simulink model "V2x_TC_IntersectionCollisionWarning_cs"  (Simulink 7.6)
settings: Solver=ode5  SolverMode=SingleTasking  StartTime=0.0  StopTime=7  FixedStep=1/100  RelTol=1e-3
PreLoadFcn:
  | check_NUTS_defined;
  | 
  | assignin('base',['model_closed_' bdroot],false);addpath('../includes_V2x');
InitFcn:
  | PreScanExperimentInitFcn(bdroot)
StopFcn:
  | PreScanExperimentStopFcn(bdroot)
undefined symbols (not defined in the file or in its folder): acceleration, actorID, actorType, DiscIntNeverNeededParam, GPS_alt, GPS_lat, GPS_long, intmax, lightBarInUse, messageID, Newton, publicActorType, Raphson, rotZ, sigbuilder_block, sireneInUse, trafficLightPriority, turnAdvice, velocity, x_ini_VRML, y_ini_VRML, yawRate, z_ini_VRML
libraries: simulink x71, PreScanSimulinkLibrary x62, PreScanUsefulBlocks x16, simulink_extras x2
(part 2 of 17)

== system V2x_TC_IntersectionCollisionWarning_cs/Antenna_Tx_7 ==
  Y : Inport
  Assignment : Assignment  IndexMode=Zero-based  SampleTime=TimeStep  IndexOptions=Starting index (dialog)  Indices=PortIndex  OutputSizes=[1 1]
  Data Store Read : DataStoreRead  DataStoreName=SimCoreActuators  SampleTime=TimeStep
  Data Store Write : DataStoreWrite  DataStoreName=SimCoreActuators
  wires:
  Assignment/1 -> Data Store Write/1
  Data Store Read/1 -> Assignment/1
  Y/1 -> Assignment/2

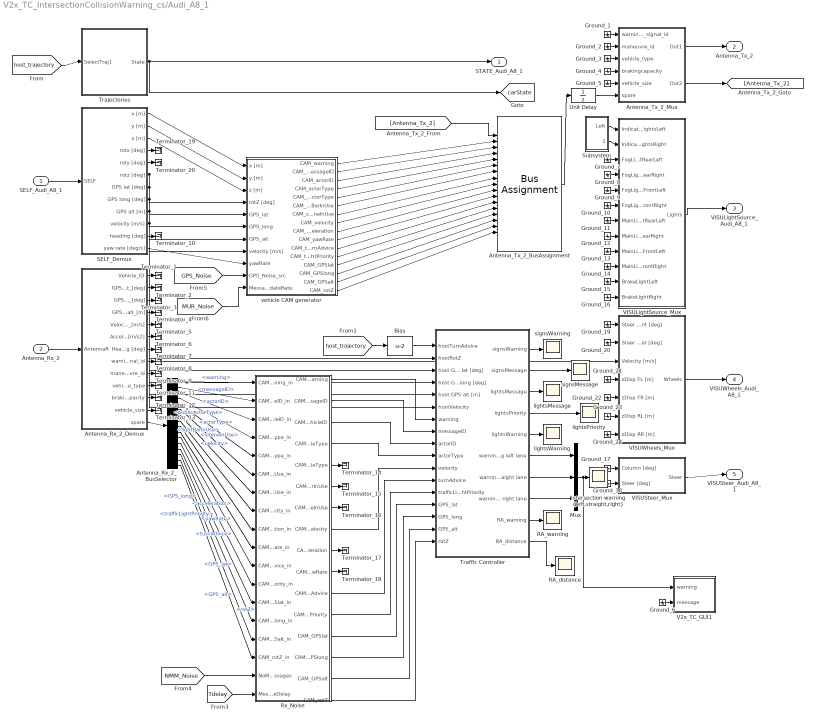
== system V2x_TC_IntersectionCollisionWarning_cs/Audi_A8_1 ==
  SELF_Audi_A8_1 : Inport  Tag=SELFInport
  Antenna_Rx_2 : Inport  Tag=AntennaRInport  Port=2
  Antenna_Rx_2_BusSelector : BusSelector  Tag=BusAntennaR  OutputSignals=warning,messageID,actorID,actorType,publicActorType,lightBarInUse,sireneInUse,velocity,acceleration,yawRate,turnAdvice,trafficLightPriority,GPS_lat,GPS_long,GPS_alt,rotZ
  Antenna_Rx_2_Demux : Subsystem  Tag=DemuxAntennaR  mask(PreScan Demux Block:){Channel=1}
      settings: DeleteFcn=PreScanBlockDeleteFcn;
      mask Display:
      | fprintf('Channel: %d',Channel)
      | port_label('output', 1, 'Vehicle_ID')
      | port_label('output', 2, 'GPS Pos Lat [deg]')
      | port_label('output', 3, 'GPS Pos Long [deg]')
      | port_label('output', 4, 'GPS Pos Alt [m]')
      | port_label('output', 5, 'Velocity [m/s]')
      | port_label('output', 6, 'Acceleration [m/s2]')
      | port_label('output', 7, 'Heading [deg]')
      | port_label('output', 8, 'Warning signal ID')
      | port_label('output', 9, 'Maneuvre ID')
      | port_label('output', 10, 'Vehicle Type')
      | port_label('output', 11, 'Braking Capacity')
      | port_label('output', 12, 'Vehicle Size')
      | port_label('output', 13, 'Spare')
  Antenna_Tx_2_BusAssignment : BusAssignment  Tag=BusAntennaT  AssignedSignals=warning,messageID,actorID,actorType,publicActorType,lightBarInUse,sireneInUse,velocity,acceleration,yawRate,turnAdvice,trafficLightPriority,GPS_lat,GPS_long,GPS_alt,rotZ
  Antenna_Tx_2_Mux : Subsystem  Tag=MuxAntennaT  mask(PreScan Mux Block:){Channel=1, TimeStep=1/100}
      mask prompts: TimeStep "Time Step [s]"
      settings: DeleteFcn=PreScanBlockDeleteFcn;
      mask Display:
      | fprintf('Channel: %d',Channel)
      | port_label('input', 1, 'Warning signal ID')
      | port_label('input', 2, 'Maneuvre ID')
      | port_label('input', 3, 'Vehicle Type')
      | port_label('input', 4, 'Braking Capacity')
      | port_label('input', 5, 'Vehicle Size')
      | port_label('input', 6, 'spare')
  Bias : Bias  Bias=-2
  Ground_{1..24} (24 alike) : Ground
  Mux : Mux  Inputs=3
  RA_distance : Scope
      settings: SaveName=ScopeData8
  RA_warning : Scope
      settings: SaveName=ScopeData7
  Rx_Noise : Subsystem
  SELF_Demux : Subsystem  Tag=DemuxSELF
      settings: DeleteFcn=PreScanBlockDeleteFcn;
      mask Type: PreScan Demux Block:
      mask Display:
      | port_label('output',1,'x [m]')
      | port_label('output',2,'y [m]')
      | port_label('output',3,'z [m]','texmode','on')
      | port_label('output',4,'Rotx [deg]','texmode','on')
      | port_label('output',5,'Roty [deg]','texmode','on')
      | port_label('output',6,'Rotz [deg]','texmode','on')
      | port_label('output',7,'GPS lat [deg]','texmode','on')
      | port_label('output',8,'GPS long [deg]','texmode','on')
      | port_label('output',9,'GPS alt [m]','texmode','on')
      | port_label('output',10,'Velocity [m/s]')
      | port_label('output',11,'Heading [deg]')
      | port_label('output',12,'Yaw rate [deg/s]')
  Subsystem : Subsystem
  Terminator_{1..20} (20 alike) : Terminator
  Traffic Controller : Subsystem
  Trajectories : Subsystem  Tag=Trajectories
      settings: DeleteFcn=PreScanBlockDeleteFcn;
      mask Type: PreScan Source Block: Trajectories
      mask Description:
      | The Trajectory block contains the trajectories defined in the PreScan Experiment Editor. The output of the trajectory block is the (vehicle) State.
      | 
      | For more information, please click Help.
      mask Help: eval('openHelp(312)')
      mask Display: image(imread('Trajectory.png'));
  Unit Delay : UnitDelay  SampleTime=-1
  V2x_TC_GUI1 : Subsystem
  VISULightSource_Mux : Subsystem  Tag=MuxLightSource
      settings: DeleteFcn=PreScanBlockDeleteFcn;
      mask Type: PreScan Mux Block:
  VISUSteer_Mux : Subsystem  Tag=MuxSteer
      settings: DeleteFcn=PreScanBlockDeleteFcn;
      mask Type: PreScan Mux Block:
  VISUWheels_Mux : Subsystem  Tag=MuxWheels  mask(PreScan Mux Block:){TimeStep=1/100}
      mask prompts: TimeStep "Auto Assigned: Time Step [s]"
      settings: DeleteFcn=PreScanBlockDeleteFcn;
  "intersection warning {left,straight,right}" : Scope
      settings: YMin=-0.1  YMax=1.1  SaveName=ScopeData5
  lightsMessage : Scope
      settings: SaveName=ScopeData2
  lightsPriority : Scope
      settings: SaveName=ScopeData3
  lightsWarning : Scope
      settings: SaveName=ScopeData4
  signsMessage : Scope
  signsWarning : Scope
  vehicle CAM generator : Subsystem
  STATE_Audi_A8_1 : Outport  Tag=StateOutport
  Antenna_Tx_2 : Outport  Tag=AntennaTOutport  Port=2
  VISULightSource_Audi_A8_1 : Outport  Tag=VisuLightsOutport  Port=3  SampleTime=1/100
  VISUWheels_Audi_A8_1 : Outport  Tag=VisuWheelsOutport  Port=4  SampleTime=1/100
  VISUSteer_Audi_A8_1 : Outport  Tag=VisuSteerOutport  Port=5  SampleTime=1/100
  tag scope: carState=global
  wires:
  [host_trajectory] -> Bias/1
  Bias/1 -> Traffic Controller/1(hostTurnAdvice)
  Mux/1 -> "intersection warning {left,straight,right}"/1, V2x_TC_GUI1/1(warning)
  Traffic Controller/1(signsWarning) -> signsWarning/1
  Traffic Controller/2(signsMessage) -> signsMessage/1
  Traffic Controller/3(lightsMessage) -> lightsMessage/1
  Traffic Controller/4(lightsPriority) -> lightsPriority/1
  Traffic Controller/5(lightsWarning) -> lightsWarning/1
  Traffic Controller/6(warning left lane) -> Mux/1
  Traffic Controller/7(warning straight lane) -> Mux/2
  Traffic Controller/8(warning right lane) -> Mux/3
  Traffic Controller/9(RA_warning) -> RA_warning/1
  Traffic Controller/10(RA_distance) -> RA_distance/1
  [NMM_Noise] -> Rx_Noise/17(NoMissedMessages)
  [Tdelay] -> Rx_Noise/18(MessageDelay)
  [GPS_Noise] -> vehicle CAM generator/10(GPS_Noise_src)
  [MUR_Noise] -> vehicle CAM generator/11(MessageUpdateRate)
  Rx_Noise/5(CAM_publicVehicleType) -> Terminator_14/1
  Rx_Noise/6(CAM_lightBarInUse) -> Terminator_15/1
  Rx_Noise/7(CAM_sireneInUse) -> Terminator_16/1
  Rx_Noise/9(CAM_acceleration) -> Terminator_17/1
  Rx_Noise/10(CAM_yawRate) -> Terminator_18/1
  Rx_Noise/1(CAM_warning) -> Traffic Controller/7(warning)
  Rx_Noise/2(CAM_messageID) -> Traffic Controller/8(messageID)
  Rx_Noise/3(CAM_vehicleID) -> Traffic Controller/9(actorID)
  Rx_Noise/4(CAM_vehicleType) -> Traffic Controller/10(actorType)
  Rx_Noise/8(CAM_velocity) -> Traffic Controller/11(velocity)
  Rx_Noise/11(CAM_turnAdvice) -> Traffic Controller/12(turnAdvice)
  Rx_Noise/12(CAM_trafficLightPriority) -> Traffic Controller/13(trafficLightPriority)
  Rx_Noise/13(CAM_GPSlat) -> Traffic Controller/14(GPS_lat)
  Rx_Noise/14(CAM_GPSlong) -> Traffic Controller/15(GPS_long)
  Rx_Noise/15(CAM_GPSalt) -> Traffic Controller/16(GPS_alt)
  Rx_Noise/16(CAM_rotZ) -> Traffic Controller/17(rotZ)
  SELF_Audi_A8_1/1 -> SELF_Demux/1(SELF)
  Antenna_Rx_2_Demux/13(spare) -> Antenna_Rx_2_BusSelector/1
  Antenna_Rx_2/1 -> Antenna_Rx_2_Demux/1(AntennaR)
  Antenna_Tx_2_Mux/2(Out2) -> [Antenna_Tx_2]
  [Antenna_Tx_2] -> Antenna_Tx_2_BusAssignment/1
  Antenna_Tx_2_Mux/1(Out1) -> Antenna_Tx_2/1
  VISULightSource_Mux/1(Lights) -> VISULightSource_Audi_A8_1/1
  VISUWheels_Mux/1(Wheels) -> VISUWheels_Audi_A8_1/1
  VISUSteer_Mux/1(Steer) -> VISUSteer_Audi_A8_1/1
  Trajectories/1(State) -> STATE_Audi_A8_1/1, [carState]
  SELF_Demux/5(roty [deg]) -> Terminator_20/1
  SELF_Demux/4(rotx [deg]) -> Terminator_19/1
  Antenna_Rx_2_Demux/12(vehicle_size) -> Terminator_13/1
  Antenna_Rx_2_Demux/11(brakingcapacity) -> Terminator_12/1
  Antenna_Rx_2_Demux/10(vehicle_type) -> Terminator_11/1
  vehicle CAM generator/16(CAM_rotZ) -> Antenna_Tx_2_BusAssignment/17
  vehicle CAM generator/15(CAM_GPSalt) -> Antenna_Tx_2_BusAssignment/16
  vehicle CAM generator/14(CAM_GPSlong) -> Antenna_Tx_2_BusAssignment/15
  vehicle CAM generator/13(CAM_GPSlat) -> Antenna_Tx_2_BusAssignment/14
  vehicle CAM generator/12(CAM_trafficLightPriority) -> Antenna_Tx_2_BusAssignment/13
  vehicle CAM generator/11(CAM_turnAdvice) -> Antenna_Tx_2_BusAssignment/12
  Antenna_Rx_2_BusSelector/16 -> Rx_Noise/16(CAM_rotZ_in)  "<rotZ>"
  Antenna_Rx_2_BusSelector/15 -> Rx_Noise/15(CAM_GPSalt_in)  "<GPS_alt>"
  Antenna_Rx_2_BusSelector/14 -> Rx_Noise/14(CAM_GPSlong_in)  "<GPS_long>"
  Antenna_Rx_2_BusSelector/13 -> Rx_Noise/13(CAM_GPSlat_in)  "<GPS_lat>"
  Antenna_Rx_2_BusSelector/12 -> Rx_Noise/12(CAM_trafficLightPriority_in)  "<trafficLightPriority>"
  Antenna_Rx_2_BusSelector/11 -> Rx_Noise/11(CAM_turnAdvice_in)  "<turnAdvice>"
  Antenna_Rx_2_BusSelector/10 -> Rx_Noise/10(CAM_yawRate_in)  "<yawRate>"
  Antenna_Rx_2_BusSelector/9 -> Rx_Noise/9(CAM_acceleration_in)  "<acceleration>"
  Antenna_Rx_2_BusSelector/8 -> Rx_Noise/8(CAM_velocity_in)  "<velocity>"
  Antenna_Rx_2_BusSelector/7 -> Rx_Noise/7(CAM_sireneInUse_in)  "<sireneInUse>"
  Antenna_Rx_2_BusSelector/6 -> Rx_Noise/6(CAM_lightBarInUse_in)  "<lightBarInUse>"
  Antenna_Rx_2_BusSelector/5 -> Rx_Noise/5(CAM_publicVehicleType_in)  "<publicActorType>"
  Antenna_Rx_2_BusSelector/4 -> Rx_Noise/4(CAM_vehicleType_in)  "<actorType>"
  Antenna_Rx_2_BusSelector/3 -> Rx_Noise/3(CAM_vehicleID_in)  "<actorID>"
  Antenna_Rx_2_BusSelector/2 -> Rx_Noise/2(CAM_messageID_in)  "<messageID>"
  Antenna_Rx_2_BusSelector/1 -> Rx_Noise/1(CAM_warning_in)  "<warning>"
  [host_trajectory] -> Trajectories/1(SelectTraj1)
  SELF_Demux/10(velocity [m/s]) -> vehicle CAM generator/8(velocity [m/s]), VISUWheels_Mux/3(Velocity [m/s]), Traffic Controller/6(hostVelocity)
  SELF_Demux/9(GPS alt [m]) -> vehicle CAM generator/7(GPS_alt), Traffic Controller/5(host GPS alt [m])
  SELF_Demux/8(GPS long [deg]) -> vehicle CAM generator/6(GPS_long), Traffic Controller/4(host GPS long [deg])
  SELF_Demux/7(GPS lat [deg]) -> vehicle CAM generator/5(GPS_lat), Traffic Controller/3(host GPS lat [deg])
  SELF_Demux/1(x [m]) -> vehicle CAM generator/1(x [m])
  SELF_Demux/12(yaw rate [deg/s]) -> vehicle CAM generator/9(yawRate)
  SELF_Demux/3(z [m]) -> vehicle CAM generator/3(z [m])
  SELF_Demux/2(y [m]) -> vehicle CAM generator/2(y [m])
  SELF_Demux/6(rotz [deg]) -> vehicle CAM generator/4(rotZ [deg]), Traffic Controller/2(hostRotZ)
  vehicle CAM generator/1(CAM_warning) -> Antenna_Tx_2_BusAssignment/2
  vehicle CAM generator/2(CAM_messageID) -> Antenna_Tx_2_BusAssignment/3
  vehicle CAM generator/3(CAM_actorID) -> Antenna_Tx_2_BusAssignment/4
  vehicle CAM generator/4(CAM_actorType) -> Antenna_Tx_2_BusAssignment/5
  vehicle CAM generator/5(CAM_publicActorType) -> Antenna_Tx_2_BusAssignment/6
  vehicle CAM generator/6(CAM_lightBarInUse) -> Antenna_Tx_2_BusAssignment/7
  vehicle CAM generator/7(CAM_sireneInUse) -> Antenna_Tx_2_BusAssignment/8
  vehicle CAM generator/8(CAM_velocity) -> Antenna_Tx_2_BusAssignment/9
  vehicle CAM generator/9(CAM_acceleration) -> Antenna_Tx_2_BusAssignment/10
  vehicle CAM generator/10(CAM_yawRate) -> Antenna_Tx_2_BusAssignment/11
  Subsystem/1(Left) -> VISULightSource_Mux/1(IndicatorLightsLeft)
  Subsystem/2(Right) -> VISULightSource_Mux/2(IndicatorLightsRight)
  Unit Delay/1 -> Antenna_Tx_2_Mux/6(spare)
  Antenna_Tx_2_BusAssignment/1 -> Unit Delay/1
  Antenna_Rx_2_Demux/1(Vehicle_ID) -> Terminator_1/1
  Antenna_Rx_2_Demux/2(GPS_pos_lat_[deg]) -> Terminator_2/1
  Antenna_Rx_2_Demux/3(GPS_pos_long_[deg]) -> Terminator_3/1
  Antenna_Rx_2_Demux/4(GPS_pos_alt_[m]) -> Terminator_4/1
  Antenna_Rx_2_Demux/5(Velocity_[m/s]) -> Terminator_5/1
  Antenna_Rx_2_Demux/6(Acceleration_[m/s2]) -> Terminator_6/1
  Antenna_Rx_2_Demux/7(Heading [deg]) -> Terminator_7/1
  Antenna_Rx_2_Demux/8(warning_signal_id) -> Terminator_8/1
  Antenna_Rx_2_Demux/9(maneuvre_id) -> Terminator_9/1
  Ground_1/1 -> Antenna_Tx_2_Mux/1(warning_signal_id)
  Ground_2/1 -> Antenna_Tx_2_Mux/2(maneuvre_id)
  Ground_3/1 -> Antenna_Tx_2_Mux/3(vehicle_type)
  Ground_4/1 -> Antenna_Tx_2_Mux/4(brakingcapacity)
  Ground_5/1 -> Antenna_Tx_2_Mux/5(vehicle_size)
  SELF_Demux/11(heading [deg]) -> Terminator_10/1
  Ground_6/1 -> V2x_TC_GUI1/2(message)
  Ground_7/1 -> VISULightSource_Mux/3(FogLightRearLeft)
  Ground_8/1 -> VISULightSource_Mux/4(FogLightRearRight)
  Ground_9/1 -> VISULightSource_Mux/5(FogLightFrontLeft)
  Ground_10/1 -> VISULightSource_Mux/6(FogLightFrontRight)
  Ground_11/1 -> VISULightSource_Mux/7(MainLightRearLeft)
  Ground_12/1 -> VISULightSource_Mux/8(MainLightRearRight)
  Ground_13/1 -> VISULightSource_Mux/9(MainLightFrontLeft)
  Ground_14/1 -> VISULightSource_Mux/10(MainLightFrontRight)
  Ground_15/1 -> VISULightSource_Mux/11(BrakeLightLeft)
  Ground_16/1 -> VISULightSource_Mux/12(BrakeLightRight)
  Ground_17/1 -> VISUSteer_Mux/1(Column [deg])
  Ground_18/1 -> VISUSteer_Mux/2(Steer [deg])
  Ground_19/1 -> VISUWheels_Mux/1(Steer Angle Front [deg])
  Ground_20/1 -> VISUWheels_Mux/2(Steer Angle Rear [deg])
  Ground_21/1 -> VISUWheels_Mux/4(zDisp FL [m])
  Ground_22/1 -> VISUWheels_Mux/5(zDisp FR [m])
  Ground_23/1 -> VISUWheels_Mux/6(zDisp RL [m])
  Ground_24/1 -> VISUWheels_Mux/7(zDisp RR [m])

== system V2x_TC_IntersectionCollisionWarning_cs/Citroen_C3_1 ==
  SELF_Citroen_C3_1 : Inport  Tag=SELFInport
  Dynamics_Empty : Subsystem  Tag=Dynamics_Empty
      mask Display: port_label('output', 1, 'State');text(0,0,' Empty\n')
  SELF_Demux : Subsystem  Tag=DemuxSELF
      settings: DeleteFcn=PreScanBlockDeleteFcn;
      mask Type: PreScan Demux Block:
      mask Display:
      | port_label('output',1,'x [m]')
      | port_label('output',2,'y [m]')
      | port_label('output',3,'z [m]','texmode','on')
      | port_label('output',4,'Rotx [deg]','texmode','on')
      | port_label('output',5,'Roty [deg]','texmode','on')
      | port_label('output',6,'Rotz [deg]','texmode','on')
      | port_label('output',7,'GPS lat [deg]','texmode','on')
      | port_label('output',8,'GPS long [deg]','texmode','on')
      | port_label('output',9,'GPS alt [m]','texmode','on')
      | port_label('output',10,'Velocity [m/s]')
      | port_label('output',11,'Heading [deg]')
      | port_label('output',12,'Yaw rate [deg/s]')
  Terminator_{1..12} (12 alike) : Terminator
  STATE_Citroen_C3_1 : Outport  Tag=StateOutport
  wires:
  SELF_Citroen_C3_1/1 -> SELF_Demux/1(SELF)
  Dynamics_Empty/1(State) -> STATE_Citroen_C3_1/1
  SELF_Demux/1(x [m]) -> Terminator_1/1
  SELF_Demux/2(y [m]) -> Terminator_2/1
  SELF_Demux/3(z [m]) -> Terminator_3/1
  SELF_Demux/4(rotx [deg]) -> Terminator_4/1
  SELF_Demux/5(roty [deg]) -> Terminator_5/1
  SELF_Demux/6(rotz [deg]) -> Terminator_6/1
  SELF_Demux/7(GPS lat [deg]) -> Terminator_7/1
  SELF_Demux/8(GPS long [deg]) -> Terminator_8/1
  SELF_Demux/9(GPS alt [m]) -> Terminator_9/1
  SELF_Demux/10(velocity [m/s]) -> Terminator_10/1
  SELF_Demux/11(heading [deg]) -> Terminator_11/1
  SELF_Demux/12(yaw rate [deg/s]) -> Terminator_12/1

== system V2x_TC_IntersectionCollisionWarning_cs/Female_Regular_wBuggy_1 ==
  SELF_Female_Regular_wBuggy_1 : Inport  Tag=SELFInport
  Dynamics_Empty : Subsystem  Tag=Dynamics_Empty
      mask Display: port_label('output', 1, 'State');text(0,0,' Empty\n')
  SELF_Demux : Subsystem  Tag=DemuxSELF
      settings: DeleteFcn=PreScanBlockDeleteFcn;
      mask Type: PreScan Demux Block:
      mask Display:
      | port_label('output',1,'x [m]')
      | port_label('output',2,'y [m]')
      | port_label('output',3,'z [m]','texmode','on')
      | port_label('output',4,'Rotx [deg]','texmode','on')
      | port_label('output',5,'Roty [deg]','texmode','on')
      | port_label('output',6,'Rotz [deg]','texmode','on')
      | port_label('output',7,'GPS lat [deg]','texmode','on')
      | port_label('output',8,'GPS long [deg]','texmode','on')
      | port_label('output',9,'GPS alt [m]','texmode','on')
      | port_label('output',10,'Velocity [m/s]')
      | port_label('output',11,'Heading [deg]')
      | port_label('output',12,'Yaw rate [deg/s]')
  Terminator_{1..12} (12 alike) : Terminator
  STATE_Female_Regular_wBuggy_1 : Outport  Tag=StateOutport
  wires:
  SELF_Female_Regular_wBuggy_1/1 -> SELF_Demux/1(SELF)
  Dynamics_Empty/1(State) -> STATE_Female_Regular_wBuggy_1/1
  SELF_Demux/1(x [m]) -> Terminator_1/1
  SELF_Demux/2(y [m]) -> Terminator_2/1
  SELF_Demux/3(z [m]) -> Terminator_3/1
  SELF_Demux/4(rotx [deg]) -> Terminator_4/1
  SELF_Demux/5(roty [deg]) -> Terminator_5/1
  SELF_Demux/6(rotz [deg]) -> Terminator_6/1
  SELF_Demux/7(GPS lat [deg]) -> Terminator_7/1
  SELF_Demux/8(GPS long [deg]) -> Terminator_8/1
  SELF_Demux/9(GPS alt [m]) -> Terminator_9/1
  SELF_Demux/10(velocity [m/s]) -> Terminator_10/1
  SELF_Demux/11(heading [deg]) -> Terminator_11/1
  SELF_Demux/12(yaw rate [deg/s]) -> Terminator_12/1

== system V2x_TC_IntersectionCollisionWarning_cs/Fiat_Bravo_1 ==
  SELF_Fiat_Bravo_1 : Inport  Tag=SELFInport
  Antenna_Rx_3 : Inport  Tag=AntennaRInport  Port=2
  Antenna_Rx_3_BusSelector : BusSelector  Tag=BusAntennaR  OutputSignals=warning,messageID,actorID,actorType,publicActorType,lightBarInUse,sireneInUse,velocity,acceleration,yawRate
  Antenna_Rx_3_Demux : Subsystem  Tag=DemuxAntennaR  mask(PreScan Demux Block:){Channel=1}
      settings: DeleteFcn=PreScanBlockDeleteFcn;
      mask Display:
      | fprintf('Channel: %d',Channel)
      | port_label('output', 1, 'Vehicle_ID')
      | port_label('output', 2, 'GPS Pos Lat [deg]')
      | port_label('output', 3, 'GPS Pos Long [deg]')
      | port_label('output', 4, 'GPS Pos Alt [m]')
      | port_label('output', 5, 'Velocity [m/s]')
      | port_label('output', 6, 'Acceleration [m/s2]')
      | port_label('output', 7, 'Heading [deg]')
      | port_label('output', 8, 'Warning signal ID')
      | port_label('output', 9, 'Maneuvre ID')
      | port_label('output', 10, 'Vehicle Type')
      | port_label('output', 11, 'Braking Capacity')
      | port_label('output', 12, 'Vehicle Size')
      | port_label('output', 13, 'Spare')
  Antenna_Tx_4_BusAssignment : BusAssignment  Tag=BusAntennaT  AssignedSignals=warning,messageID,actorID,actorType,publicActorType,lightBarInUse,sireneInUse,velocity,acceleration,yawRate,turnAdvice,trafficLightPriority,GPS_lat,GPS_long,GPS_alt,rotZ
  Antenna_Tx_4_Mux : Subsystem  Tag=MuxAntennaT  mask(PreScan Mux Block:){Channel=1, TimeStep=1/100}
      mask prompts: TimeStep "Time Step [s]"
      settings: DeleteFcn=PreScanBlockDeleteFcn;
      mask Display:
      | fprintf('Channel: %d',Channel)
      | port_label('input', 1, 'Warning signal ID')
      | port_label('input', 2, 'Maneuvre ID')
      | port_label('input', 3, 'Vehicle Type')
      | port_label('input', 4, 'Braking Capacity')
      | port_label('input', 5, 'Vehicle Size')
      | port_label('input', 6, 'spare')
  Dynamics_Empty : Subsystem  Tag=Dynamics_Empty
      mask Display: port_label('output', 1, 'State');text(0,0,' Empty\n')
  Ground_{1..5} (5 alike) : Ground
  SELF_Demux : Subsystem  Tag=DemuxSELF
      settings: DeleteFcn=PreScanBlockDeleteFcn;
      mask Type: PreScan Demux Block:
      mask Display:
      | port_label('output',1,'x [m]')
      | port_label('output',2,'y [m]')
      | port_label('output',3,'z [m]','texmode','on')
      | port_label('output',4,'Rotx [deg]','texmode','on')
      | port_label('output',5,'Roty [deg]','texmode','on')
      | port_label('output',6,'Rotz [deg]','texmode','on')
      | port_label('output',7,'GPS lat [deg]','texmode','on')
      | port_label('output',8,'GPS long [deg]','texmode','on')
      | port_label('output',9,'GPS alt [m]','texmode','on')
      | port_label('output',10,'Velocity [m/s]')
      | port_label('output',11,'Heading [deg]')
      | port_label('output',12,'Yaw rate [deg/s]')
  Terminator_{1..25} (25 alike) : Terminator
  Unit Delay : UnitDelay  SampleTime=-1
  vehicle CAM generator : Subsystem
  STATE_Fiat_Bravo_1 : Outport  Tag=StateOutport
  Antenna_Tx_4 : Outport  Tag=AntennaTOutport  Port=2
  wires:
  [GPS_Noise] -> vehicle CAM generator/10(GPS_Noise_src)
  [MUR_Noise] -> vehicle CAM generator/11(MessageUpdateRate)
  SELF_Fiat_Bravo_1/1 -> SELF_Demux/1(SELF)
  Antenna_Rx_3_Demux/13(spare) -> Antenna_Rx_3_BusSelector/1
  Antenna_Rx_3/1 -> Antenna_Rx_3_Demux/1(AntennaR)
  Antenna_Tx_4_Mux/2(Out2) -> [Antenna_Tx_4]
  [Antenna_Tx_4] -> Antenna_Tx_4_BusAssignment/1
  Antenna_Tx_4_Mux/1(Out1) -> Antenna_Tx_4/1
  Dynamics_Empty/1(State) -> STATE_Fiat_Bravo_1/1
  vehicle CAM generator/16(CAM_rotZ) -> Antenna_Tx_4_BusAssignment/17
  vehicle CAM generator/15(CAM_GPSalt) -> Antenna_Tx_4_BusAssignment/16
  vehicle CAM generator/14(CAM_GPSlong) -> Antenna_Tx_4_BusAssignment/15
  vehicle CAM generator/13(CAM_GPSlat) -> Antenna_Tx_4_BusAssignment/14
  vehicle CAM generator/12(CAM_trafficLightPriority) -> Antenna_Tx_4_BusAssignment/13
  vehicle CAM generator/11(CAM_turnAdvice) -> Antenna_Tx_4_BusAssignment/12
  SELF_Demux/12(yaw rate [deg/s]) -> vehicle CAM generator/9(yawRate)
  SELF_Demux/10(velocity [m/s]) -> vehicle CAM generator/8(velocity [m/s])
  SELF_Demux/9(GPS alt [m]) -> vehicle CAM generator/7(GPS_alt)
  SELF_Demux/8(GPS long [deg]) -> vehicle CAM generator/6(GPS_long)
  SELF_Demux/7(GPS lat [deg]) -> vehicle CAM generator/5(GPS_lat)
  SELF_Demux/3(z [m]) -> vehicle CAM generator/3(z [m])
  SELF_Demux/2(y [m]) -> vehicle CAM generator/2(y [m])
  SELF_Demux/1(x [m]) -> vehicle CAM generator/1(x [m])
  SELF_Demux/6(rotz [deg]) -> vehicle CAM generator/4(rotZ [deg])
  vehicle CAM generator/1(CAM_warning) -> Antenna_Tx_4_BusAssignment/2
  vehicle CAM generator/2(CAM_messageID) -> Antenna_Tx_4_BusAssignment/3
  vehicle CAM generator/3(CAM_actorID) -> Antenna_Tx_4_BusAssignment/4
  vehicle CAM generator/4(CAM_actorType) -> Antenna_Tx_4_BusAssignment/5
  vehicle CAM generator/5(CAM_publicActorType) -> Antenna_Tx_4_BusAssignment/6
  vehicle CAM generator/6(CAM_lightBarInUse) -> Antenna_Tx_4_BusAssignment/7
  vehicle CAM generator/7(CAM_sireneInUse) -> Antenna_Tx_4_BusAssignment/8
  vehicle CAM generator/8(CAM_velocity) -> Antenna_Tx_4_BusAssignment/9
  vehicle CAM generator/9(CAM_acceleration) -> Antenna_Tx_4_BusAssignment/10
  vehicle CAM generator/10(CAM_yawRate) -> Antenna_Tx_4_BusAssignment/11
  Antenna_Rx_3_BusSelector/1 -> Terminator_16/1  "<warning>"
  Antenna_Rx_3_BusSelector/2 -> Terminator_17/1  "<messageID>"
  Antenna_Rx_3_BusSelector/3 -> Terminator_18/1  "<actorID>"
  Antenna_Rx_3_BusSelector/4 -> Terminator_19/1  "<actorType>"
  Antenna_Rx_3_BusSelector/5 -> Terminator_20/1  "<publicActorType>"
  Antenna_Rx_3_BusSelector/6 -> Terminator_21/1  "<lightBarInUse>"
  Antenna_Rx_3_BusSelector/7 -> Terminator_22/1  "<sireneInUse>"
  Antenna_Rx_3_BusSelector/8 -> Terminator_23/1  "<velocity>"
  Antenna_Rx_3_BusSelector/9 -> Terminator_24/1  "<acceleration>"
  Antenna_Rx_3_BusSelector/10 -> Terminator_25/1  "<yawRate>"
  Unit Delay/1 -> Antenna_Tx_4_Mux/6(spare)
  Antenna_Tx_4_BusAssignment/1 -> Unit Delay/1
  Antenna_Rx_3_Demux/1(Vehicle_ID) -> Terminator_1/1
  Antenna_Rx_3_Demux/2(GPS_pos_lat_[deg]) -> Terminator_2/1
  Antenna_Rx_3_Demux/3(GPS_pos_long_[deg]) -> Terminator_3/1
  Antenna_Rx_3_Demux/4(GPS_pos_alt_[m]) -> Terminator_4/1
  Antenna_Rx_3_Demux/5(Velocity_[m/s]) -> Terminator_5/1
  Antenna_Rx_3_Demux/6(Acceleration_[m/s2]) -> Terminator_6/1
  Antenna_Rx_3_Demux/7(Heading [deg]) -> Terminator_7/1
  Antenna_Rx_3_Demux/8(warning_signal_id) -> Terminator_8/1
  Antenna_Rx_3_Demux/9(maneuvre_id) -> Terminator_9/1
  Antenna_Rx_3_Demux/10(vehicle_type) -> Terminator_10/1
  Antenna_Rx_3_Demux/11(brakingcapacity) -> Terminator_11/1
  Antenna_Rx_3_Demux/12(vehicle_size) -> Terminator_12/1
  Ground_1/1 -> Antenna_Tx_4_Mux/1(warning_signal_id)
  Ground_2/1 -> Antenna_Tx_4_Mux/2(maneuvre_id)
  Ground_3/1 -> Antenna_Tx_4_Mux/3(vehicle_type)
  Ground_4/1 -> Antenna_Tx_4_Mux/4(brakingcapacity)
  Ground_5/1 -> Antenna_Tx_4_Mux/5(vehicle_size)
  SELF_Demux/4(rotx [deg]) -> Terminator_13/1
  SELF_Demux/5(roty [deg]) -> Terminator_14/1
  SELF_Demux/11(heading [deg]) -> Terminator_15/1

== system V2x_TC_IntersectionCollisionWarning_cs/Ford_Fiesta_1 ==
  SELF_Ford_Fiesta_1 : Inport  Tag=SELFInport
  Dynamics_Empty : Subsystem  Tag=Dynamics_Empty
      mask Display: port_label('output', 1, 'State');text(0,0,' Empty\n')
  SELF_Demux : Subsystem  Tag=DemuxSELF
      settings: DeleteFcn=PreScanBlockDeleteFcn;
      mask Type: PreScan Demux Block:
      mask Display:
      | port_label('output',1,'x [m]')
      | port_label('output',2,'y [m]')
      | port_label('output',3,'z [m]','texmode','on')
      | port_label('output',4,'Rotx [deg]','texmode','on')
      | port_label('output',5,'Roty [deg]','texmode','on')
      | port_label('output',6,'Rotz [deg]','texmode','on')
      | port_label('output',7,'GPS lat [deg]','texmode','on')
      | port_label('output',8,'GPS long [deg]','texmode','on')
      | port_label('output',9,'GPS alt [m]','texmode','on')
      | port_label('output',10,'Velocity [m/s]')
      | port_label('output',11,'Heading [deg]')
      | port_label('output',12,'Yaw rate [deg/s]')
  Terminator_{1..12} (12 alike) : Terminator
  STATE_Ford_Fiesta_1 : Outport  Tag=StateOutport
  wires:
  SELF_Ford_Fiesta_1/1 -> SELF_Demux/1(SELF)
  Dynamics_Empty/1(State) -> STATE_Ford_Fiesta_1/1
  SELF_Demux/1(x [m]) -> Terminator_1/1
  SELF_Demux/2(y [m]) -> Terminator_2/1
  SELF_Demux/3(z [m]) -> Terminator_3/1
  SELF_Demux/4(rotx [deg]) -> Terminator_4/1
  SELF_Demux/5(roty [deg]) -> Terminator_5/1
  SELF_Demux/6(rotz [deg]) -> Terminator_6/1
  SELF_Demux/7(GPS lat [deg]) -> Terminator_7/1
  SELF_Demux/8(GPS long [deg]) -> Terminator_8/1
  SELF_Demux/9(GPS alt [m]) -> Terminator_9/1
  SELF_Demux/10(velocity [m/s]) -> Terminator_10/1
  SELF_Demux/11(heading [deg]) -> Terminator_11/1
  SELF_Demux/12(yaw rate [deg/s]) -> Terminator_12/1

== system V2x_TC_IntersectionCollisionWarning_cs/Ford_FocusWagon_1 ==
  SELF_Ford_FocusWagon_1 : Inport  Tag=SELFInport
  Dynamics_Empty : Subsystem  Tag=Dynamics_Empty
      mask Display: port_label('output', 1, 'State');text(0,0,' Empty\n')
  SELF_Demux : Subsystem  Tag=DemuxSELF
      settings: DeleteFcn=PreScanBlockDeleteFcn;
      mask Type: PreScan Demux Block:
      mask Display:
      | port_label('output',1,'x [m]')
      | port_label('output',2,'y [m]')
      | port_label('output',3,'z [m]','texmode','on')
      | port_label('output',4,'Rotx [deg]','texmode','on')
      | port_label('output',5,'Roty [deg]','texmode','on')
      | port_label('output',6,'Rotz [deg]','texmode','on')
      | port_label('output',7,'GPS lat [deg]','texmode','on')
      | port_label('output',8,'GPS long [deg]','texmode','on')
      | port_label('output',9,'GPS alt [m]','texmode','on')
      | port_label('output',10,'Velocity [m/s]')
      | port_label('output',11,'Heading [deg]')
      | port_label('output',12,'Yaw rate [deg/s]')
  Terminator_{1..12} (12 alike) : Terminator
  STATE_Ford_FocusWagon_1 : Outport  Tag=StateOutport
  wires:
  SELF_Ford_FocusWagon_1/1 -> SELF_Demux/1(SELF)
  Dynamics_Empty/1(State) -> STATE_Ford_FocusWagon_1/1
  SELF_Demux/1(x [m]) -> Terminator_1/1
  SELF_Demux/2(y [m]) -> Terminator_2/1
  SELF_Demux/3(z [m]) -> Terminator_3/1
  SELF_Demux/4(rotx [deg]) -> Terminator_4/1
  SELF_Demux/5(roty [deg]) -> Terminator_5/1
  SELF_Demux/6(rotz [deg]) -> Terminator_6/1
  SELF_Demux/7(GPS lat [deg]) -> Terminator_7/1
  SELF_Demux/8(GPS long [deg]) -> Terminator_8/1
  SELF_Demux/9(GPS alt [m]) -> Terminator_9/1
  SELF_Demux/10(velocity [m/s]) -> Terminator_10/1
  SELF_Demux/11(heading [deg]) -> Terminator_11/1
  SELF_Demux/12(yaw rate [deg/s]) -> Terminator_12/1

== system V2x_TC_IntersectionCollisionWarning_cs/GetSensors ==
  dummy : Inport
  GetSensors : M-S-Function  FunctionName=GetSensorsBlock  Parameters=TimeStep NrOfSensors
  tag scope: SimCoreSensors=global
  wires:
  GetSensors/1 -> [SimCoreSensors]
  dummy/1 -> GetSensors/1

== system V2x_TC_IntersectionCollisionWarning_cs/Male_Regular_wSuitcase_1 ==
  SELF_Male_Regular_wSuitcase_1 : Inport  Tag=SELFInport
  Dynamics_Empty : Subsystem  Tag=Dynamics_Empty
      mask Display: port_label('output', 1, 'State');text(0,0,' Empty\n')
  SELF_Demux : Subsystem  Tag=DemuxSELF
      settings: DeleteFcn=PreScanBlockDeleteFcn;
      mask Type: PreScan Demux Block:
      mask Display:
      | port_label('output',1,'x [m]')
      | port_label('output',2,'y [m]')
      | port_label('output',3,'z [m]','texmode','on')
      | port_label('output',4,'Rotx [deg]','texmode','on')
      | port_label('output',5,'Roty [deg]','texmode','on')
      | port_label('output',6,'Rotz [deg]','texmode','on')
      | port_label('output',7,'GPS lat [deg]','texmode','on')
      | port_label('output',8,'GPS long [deg]','texmode','on')
      | port_label('output',9,'GPS alt [m]','texmode','on')
      | port_label('output',10,'Velocity [m/s]')
      | port_label('output',11,'Heading [deg]')
      | port_label('output',12,'Yaw rate [deg/s]')
  Terminator_{1..12} (12 alike) : Terminator
  STATE_Male_Regular_wSuitcase_1 : Outport  Tag=StateOutport
  wires:
  SELF_Male_Regular_wSuitcase_1/1 -> SELF_Demux/1(SELF)
  Dynamics_Empty/1(State) -> STATE_Male_Regular_wSuitcase_1/1
  SELF_Demux/1(x [m]) -> Terminator_1/1
  SELF_Demux/2(y [m]) -> Terminator_2/1
  SELF_Demux/3(z [m]) -> Terminator_3/1
  SELF_Demux/4(rotx [deg]) -> Terminator_4/1
  SELF_Demux/5(roty [deg]) -> Terminator_5/1
  SELF_Demux/6(rotz [deg]) -> Terminator_6/1
  SELF_Demux/7(GPS lat [deg]) -> Terminator_7/1
  SELF_Demux/8(GPS long [deg]) -> Terminator_8/1
  SELF_Demux/9(GPS alt [m]) -> Terminator_9/1
  SELF_Demux/10(velocity [m/s]) -> Terminator_10/1
  SELF_Demux/11(heading [deg]) -> Terminator_11/1
  SELF_Demux/12(yaw rate [deg/s]) -> Terminator_12/1

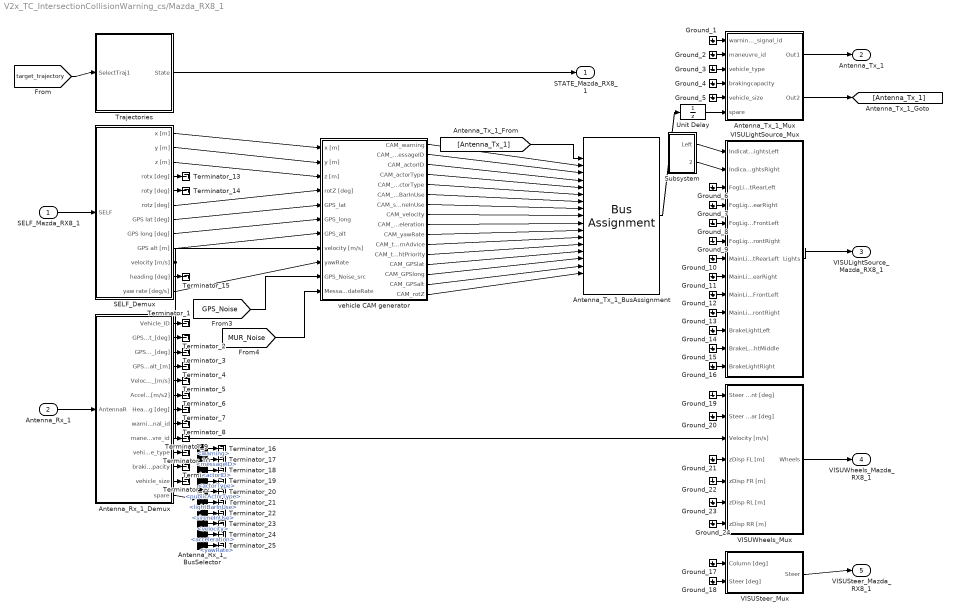
== system V2x_TC_IntersectionCollisionWarning_cs/Mazda_RX8_1 ==
  SELF_Mazda_RX8_1 : Inport  Tag=SELFInport
  Antenna_Rx_1 : Inport  Tag=AntennaRInport  Port=2
  Antenna_Rx_1_BusSelector : BusSelector  Tag=BusAntennaR  OutputSignals=warning,messageID,actorID,actorType,publicActorType,lightBarInUse,sireneInUse,velocity,acceleration,yawRate
  Antenna_Rx_1_Demux : Subsystem  Tag=DemuxAntennaR  mask(PreScan Demux Block:){Channel=1}
      settings: DeleteFcn=PreScanBlockDeleteFcn;
      mask Display:
      | fprintf('Channel: %d',Channel)
      | port_label('output', 1, 'Vehicle_ID')
      | port_label('output', 2, 'GPS Pos Lat [deg]')
      | port_label('output', 3, 'GPS Pos Long [deg]')
      | port_label('output', 4, 'GPS Pos Alt [m]')
      | port_label('output', 5, 'Velocity [m/s]')
      | port_label('output', 6, 'Acceleration [m/s2]')
      | port_label('output', 7, 'Heading [deg]')
      | port_label('output', 8, 'Warning signal ID')
      | port_label('output', 9, 'Maneuvre ID')
      | port_label('output', 10, 'Vehicle Type')
      | port_label('output', 11, 'Braking Capacity')
      | port_label('output', 12, 'Vehicle Size')
      | port_label('output', 13, 'Spare')
  Antenna_Tx_1_BusAssignment : BusAssignment  Tag=BusAntennaT  AssignedSignals=warning,messageID,actorID,actorType,publicActorType,lightBarInUse,sireneInUse,velocity,acceleration,yawRate,turnAdvice,trafficLightPriority,GPS_lat,GPS_long,GPS_alt,rotZ
  Antenna_Tx_1_Mux : Subsystem  Tag=MuxAntennaT  mask(PreScan Mux Block:){Channel=1, TimeStep=1/100}
      mask prompts: TimeStep "Time Step [s]"
      settings: DeleteFcn=PreScanBlockDeleteFcn;
      mask Display:
      | fprintf('Channel: %d',Channel)
      | port_label('input', 1, 'Warning signal ID')
      | port_label('input', 2, 'Maneuvre ID')
      | port_label('input', 3, 'Vehicle Type')
      | port_label('input', 4, 'Braking Capacity')
      | port_label('input', 5, 'Vehicle Size')
      | port_label('input', 6, 'spare')
  Ground_{1..24} (24 alike) : Ground
  SELF_Demux : Subsystem  Tag=DemuxSELF
      settings: DeleteFcn=PreScanBlockDeleteFcn;
      mask Type: PreScan Demux Block:
      mask Display:
      | port_label('output',1,'x [m]')
      | port_label('output',2,'y [m]')
      | port_label('output',3,'z [m]','texmode','on')
      | port_label('output',4,'Rotx [deg]','texmode','on')
      | port_label('output',5,'Roty [deg]','texmode','on')
      | port_label('output',6,'Rotz [deg]','texmode','on')
      | port_label('output',7,'GPS lat [deg]','texmode','on')
      | port_label('output',8,'GPS long [deg]','texmode','on')
      | port_label('output',9,'GPS alt [m]','texmode','on')
      | port_label('output',10,'Velocity [m/s]')
      | port_label('output',11,'Heading [deg]')
      | port_label('output',12,'Yaw rate [deg/s]')
  Subsystem : Subsystem
  Terminator_{1..25} (25 alike) : Terminator
  Trajectories : Subsystem  Tag=Trajectories
      settings: DeleteFcn=PreScanBlockDeleteFcn;
      mask Type: PreScan Source Block: Trajectories
      mask Description:
      | The Trajectory block contains the trajectories defined in the PreScan Experiment Editor. The output of the trajectory block is the (vehicle) State.
      | 
      | For more information, please click Help.
      mask Help: eval('openHelp(312)')
      mask Display: image(imread('Trajectory.png'));
  Unit Delay : UnitDelay  SampleTime=-1
  VISULightSource_Mux : Subsystem  Tag=MuxLightSource
      settings: DeleteFcn=PreScanBlockDeleteFcn;
      mask Type: PreScan Mux Block:
  VISUSteer_Mux : Subsystem  Tag=MuxSteer
      settings: DeleteFcn=PreScanBlockDeleteFcn;
      mask Type: PreScan Mux Block:
  VISUWheels_Mux : Subsystem  Tag=MuxWheels  mask(PreScan Mux Block:){TimeStep=1/100}
      mask prompts: TimeStep "Auto Assigned: Time Step [s]"
      settings: DeleteFcn=PreScanBlockDeleteFcn;
  vehicle CAM generator : Subsystem
  STATE_Mazda_RX8_1 : Outport  Tag=StateOutport
  Antenna_Tx_1 : Outport  Tag=AntennaTOutport  Port=2
  VISULightSource_Mazda_RX8_1 : Outport  Tag=VisuLightsOutport  Port=3  SampleTime=1/100
  VISUWheels_Mazda_RX8_1 : Outport  Tag=VisuWheelsOutport  Port=4  SampleTime=1/100
  VISUSteer_Mazda_RX8_1 : Outport  Tag=VisuSteerOutport  Port=5  SampleTime=1/100
  wires:
  [GPS_Noise] -> vehicle CAM generator/10(GPS_Noise_src)
  [MUR_Noise] -> vehicle CAM generator/11(MessageUpdateRate)
  SELF_Mazda_RX8_1/1 -> SELF_Demux/1(SELF)
  Antenna_Rx_1_Demux/13(spare) -> Antenna_Rx_1_BusSelector/1
  Antenna_Rx_1/1 -> Antenna_Rx_1_Demux/1(AntennaR)
  Antenna_Tx_1_Mux/2(Out2) -> [Antenna_Tx_1]
  [Antenna_Tx_1] -> Antenna_Tx_1_BusAssignment/1
  Antenna_Tx_1_Mux/1(Out1) -> Antenna_Tx_1/1
  VISULightSource_Mux/1(Lights) -> VISULightSource_Mazda_RX8_1/1
  VISUWheels_Mux/1(Wheels) -> VISUWheels_Mazda_RX8_1/1
  VISUSteer_Mux/1(Steer) -> VISUSteer_Mazda_RX8_1/1
  Trajectories/1(State) -> STATE_Mazda_RX8_1/1
  vehicle CAM generator/16(CAM_rotZ) -> Antenna_Tx_1_BusAssignment/17
  vehicle CAM generator/15(CAM_GPSalt) -> Antenna_Tx_1_BusAssignment/16
  vehicle CAM generator/14(CAM_GPSlong) -> Antenna_Tx_1_BusAssignment/15
  vehicle CAM generator/13(CAM_GPSlat) -> Antenna_Tx_1_BusAssignment/14
  vehicle CAM generator/12(CAM_trafficLightPriority) -> Antenna_Tx_1_BusAssignment/13
  vehicle CAM generator/11(CAM_turnAdvice) -> Antenna_Tx_1_BusAssignment/12
  SELF_Demux/12(yaw rate [deg/s]) -> vehicle CAM generator/9(yawRate)
  SELF_Demux/10(velocity [m/s]) -> vehicle CAM generator/8(velocity [m/s]), VISUWheels_Mux/3(Velocity [m/s])
  SELF_Demux/9(GPS alt [m]) -> vehicle CAM generator/7(GPS_alt)
  SELF_Demux/8(GPS long [deg]) -> vehicle CAM generator/6(GPS_long)
  SELF_Demux/7(GPS lat [deg]) -> vehicle CAM generator/5(GPS_lat)
  SELF_Demux/6(rotz [deg]) -> vehicle CAM generator/4(rotZ [deg])
  SELF_Demux/3(z [m]) -> vehicle CAM generator/3(z [m])
  SELF_Demux/2(y [m]) -> vehicle CAM generator/2(y [m])
  SELF_Demux/1(x [m]) -> vehicle CAM generator/1(x [m])
  [target_trajectory] -> Trajectories/1(SelectTraj1)
  vehicle CAM generator/1(CAM_warning) -> Antenna_Tx_1_BusAssignment/2
  vehicle CAM generator/2(CAM_messageID) -> Antenna_Tx_1_BusAssignment/3
  vehicle CAM generator/3(CAM_actorID) -> Antenna_Tx_1_BusAssignment/4
  vehicle CAM generator/4(CAM_actorType) -> Antenna_Tx_1_BusAssignment/5
  vehicle CAM generator/5(CAM_publicActorType) -> Antenna_Tx_1_BusAssignment/6
  vehicle CAM generator/6(CAM_lightBarInUse) -> Antenna_Tx_1_BusAssignment/7
  vehicle CAM generator/7(CAM_sireneInUse) -> Antenna_Tx_1_BusAssignment/8
  vehicle CAM generator/8(CAM_velocity) -> Antenna_Tx_1_BusAssignment/9
  vehicle CAM generator/9(CAM_acceleration) -> Antenna_Tx_1_BusAssignment/10
  vehicle CAM generator/10(CAM_yawRate) -> Antenna_Tx_1_BusAssignment/11
  Antenna_Rx_1_BusSelector/1 -> Terminator_16/1  "<warning>"
  Antenna_Rx_1_BusSelector/2 -> Terminator_17/1  "<messageID>"
  Antenna_Rx_1_BusSelector/3 -> Terminator_18/1  "<actorID>"
  Antenna_Rx_1_BusSelector/4 -> Terminator_19/1  "<actorType>"
  Antenna_Rx_1_BusSelector/5 -> Terminator_20/1  "<publicActorType>"
  Antenna_Rx_1_BusSelector/6 -> Terminator_21/1  "<lightBarInUse>"
  Antenna_Rx_1_BusSelector/7 -> Terminator_22/1  "<sireneInUse>"
  Antenna_Rx_1_BusSelector/8 -> Terminator_23/1  "<velocity>"
  Antenna_Rx_1_BusSelector/9 -> Terminator_24/1  "<acceleration>"
  Antenna_Rx_1_BusSelector/10 -> Terminator_25/1  "<yawRate>"
  Subsystem/2(Right) -> VISULightSource_Mux/2(IndicatorLightsRight)
  Subsystem/1(Left) -> VISULightSource_Mux/1(IndicatorLightsLeft)
  Unit Delay/1 -> Antenna_Tx_1_Mux/6(spare)
  Antenna_Tx_1_BusAssignment/1 -> Unit Delay/1
  Antenna_Rx_1_Demux/1(Vehicle_ID) -> Terminator_1/1
  Antenna_Rx_1_Demux/2(GPS_pos_lat_[deg]) -> Terminator_2/1
  Antenna_Rx_1_Demux/3(GPS_pos_long_[deg]) -> Terminator_3/1
  Antenna_Rx_1_Demux/4(GPS_pos_alt_[m]) -> Terminator_4/1
  Antenna_Rx_1_Demux/5(Velocity_[m/s]) -> Terminator_5/1
  Antenna_Rx_1_Demux/6(Acceleration_[m/s2]) -> Terminator_6/1
  Antenna_Rx_1_Demux/7(Heading [deg]) -> Terminator_7/1
  Antenna_Rx_1_Demux/8(warning_signal_id) -> Terminator_8/1
  Antenna_Rx_1_Demux/9(maneuvre_id) -> Terminator_9/1
  Antenna_Rx_1_Demux/10(vehicle_type) -> Terminator_10/1
  Antenna_Rx_1_Demux/11(brakingcapacity) -> Terminator_11/1
  Antenna_Rx_1_Demux/12(vehicle_size) -> Terminator_12/1
  Ground_1/1 -> Antenna_Tx_1_Mux/1(warning_signal_id)
  Ground_2/1 -> Antenna_Tx_1_Mux/2(maneuvre_id)
  Ground_3/1 -> Antenna_Tx_1_Mux/3(vehicle_type)
  Ground_4/1 -> Antenna_Tx_1_Mux/4(brakingcapacity)
  Ground_5/1 -> Antenna_Tx_1_Mux/5(vehicle_size)
  SELF_Demux/4(rotx [deg]) -> Terminator_13/1
  SELF_Demux/5(roty [deg]) -> Terminator_14/1
  SELF_Demux/11(heading [deg]) -> Terminator_15/1
  Ground_6/1 -> VISULightSource_Mux/3(FogLightRearLeft)
  Ground_7/1 -> VISULightSource_Mux/4(FogLightRearRight)
  Ground_8/1 -> VISULightSource_Mux/5(FogLightFrontLeft)
  Ground_9/1 -> VISULightSource_Mux/6(FogLightFrontRight)
  Ground_10/1 -> VISULightSource_Mux/7(MainLightRearLeft)
  Ground_11/1 -> VISULightSource_Mux/8(MainLightRearRight)
  Ground_12/1 -> VISULightSource_Mux/9(MainLightFrontLeft)
  Ground_13/1 -> VISULightSource_Mux/10(MainLightFrontRight)
  Ground_14/1 -> VISULightSource_Mux/11(BrakeLightLeft)
  Ground_15/1 -> VISULightSource_Mux/12(BrakeLightMiddle)
  Ground_16/1 -> VISULightSource_Mux/13(BrakeLightRight)
  Ground_17/1 -> VISUSteer_Mux/1(Column [deg])
  Ground_18/1 -> VISUSteer_Mux/2(Steer [deg])
  Ground_19/1 -> VISUWheels_Mux/1(Steer Angle Front [deg])
  Ground_20/1 -> VISUWheels_Mux/2(Steer Angle Rear [deg])
  Ground_21/1 -> VISUWheels_Mux/4(zDisp FL [m])
  Ground_22/1 -> VISUWheels_Mux/5(zDisp FR [m])
  Ground_23/1 -> VISUWheels_Mux/6(zDisp RL [m])
  Ground_24/1 -> VISUWheels_Mux/7(zDisp RR [m])

== system V2x_TC_IntersectionCollisionWarning_cs/Mazda_RX8_2 ==
  SELF_Mazda_RX8_2 : Inport  Tag=SELFInport
  Dynamics_Empty : Subsystem  Tag=Dynamics_Empty
      mask Display: port_label('output', 1, 'State');text(0,0,' Empty\n')
  SELF_Demux : Subsystem  Tag=DemuxSELF
      settings: DeleteFcn=PreScanBlockDeleteFcn;
      mask Type: PreScan Demux Block:
      mask Display:
      | port_label('output',1,'x [m]')
      | port_label('output',2,'y [m]')
      | port_label('output',3,'z [m]','texmode','on')
      | port_label('output',4,'Rotx [deg]','texmode','on')
      | port_label('output',5,'Roty [deg]','texmode','on')
      | port_label('output',6,'Rotz [deg]','texmode','on')
      | port_label('output',7,'GPS lat [deg]','texmode','on')
      | port_label('output',8,'GPS long [deg]','texmode','on')
      | port_label('output',9,'GPS alt [m]','texmode','on')
      | port_label('output',10,'Velocity [m/s]')
      | port_label('output',11,'Heading [deg]')
      | port_label('output',12,'Yaw rate [deg/s]')
  Terminator_{1..12} (12 alike) : Terminator
  STATE_Mazda_RX8_2 : Outport  Tag=StateOutport
  wires:
  SELF_Mazda_RX8_2/1 -> SELF_Demux/1(SELF)
  Dynamics_Empty/1(State) -> STATE_Mazda_RX8_2/1
  SELF_Demux/1(x [m]) -> Terminator_1/1
  SELF_Demux/2(y [m]) -> Terminator_2/1
  SELF_Demux/3(z [m]) -> Terminator_3/1
  SELF_Demux/4(rotx [deg]) -> Terminator_4/1
  SELF_Demux/5(roty [deg]) -> Terminator_5/1
  SELF_Demux/6(rotz [deg]) -> Terminator_6/1
  SELF_Demux/7(GPS lat [deg]) -> Terminator_7/1
  SELF_Demux/8(GPS long [deg]) -> Terminator_8/1
  SELF_Demux/9(GPS alt [m]) -> Terminator_9/1
  SELF_Demux/10(velocity [m/s]) -> Terminator_10/1
  SELF_Demux/11(heading [deg]) -> Terminator_11/1
  SELF_Demux/12(yaw rate [deg/s]) -> Terminator_12/1

== system V2x_TC_IntersectionCollisionWarning_cs/Noise generator ==
  DISPLAY : M-S-Function  FunctionName=msfcn_V2X_PlotSpeed
  Scope1 : Scope  NumInputPorts=4
      settings: YMin=-5~-5~-5~-5  YMax=5~5~5~5
  tag scope: GPS_Noise=global, MUR_Noise=global, NMM_Noise=global, Tdelay=global
  wires:
  DISPLAY/1 -> Scope1/1, [GPS_Noise]
  DISPLAY/2 -> Scope1/2, [MUR_Noise]
  DISPLAY/3 -> Scope1/3, [NMM_Noise]
  DISPLAY/4 -> Scope1/4, [Tdelay]

== system V2x_TC_IntersectionCollisionWarning_cs/SELF_Actros_2541_1 ==
  Selector : Selector  IndexMode=Zero-based  IndexOptions=Starting index (dialog)  Indices=PortIndex  OutputSizes=PortWidth(1)
  U : Outport
  wires:
  Selector/1 -> U/1
  [SimCoreSensors] -> Selector/1

== system V2x_TC_IntersectionCollisionWarning_cs/SELF_Audi_A8_1 ==
  Selector : Selector  IndexMode=Zero-based  IndexOptions=Starting index (dialog)  Indices=PortIndex  OutputSizes=PortWidth(1)
  U : Outport
  wires:
  Selector/1 -> U/1
  [SimCoreSensors] -> Selector/1

== system V2x_TC_IntersectionCollisionWarning_cs/SELF_Citroen_C3_1 ==
  Selector : Selector  IndexMode=Zero-based  IndexOptions=Starting index (dialog)  Indices=PortIndex  OutputSizes=PortWidth(1)
  U : Outport
  wires:
  Selector/1 -> U/1
  [SimCoreSensors] -> Selector/1

== system V2x_TC_IntersectionCollisionWarning_cs/SELF_Female_Regular_wBuggy_1 ==
  Selector : Selector  IndexMode=Zero-based  IndexOptions=Starting index (dialog)  Indices=PortIndex  OutputSizes=PortWidth(1)
  U : Outport
  wires:
  Selector/1 -> U/1
  [SimCoreSensors] -> Selector/1

== system V2x_TC_IntersectionCollisionWarning_cs/SELF_Fiat_Bravo_1 ==
  Selector : Selector  IndexMode=Zero-based  IndexOptions=Starting index (dialog)  Indices=PortIndex  OutputSizes=PortWidth(1)
  U : Outport
  wires:
  Selector/1 -> U/1
  [SimCoreSensors] -> Selector/1

== system V2x_TC_IntersectionCollisionWarning_cs/SELF_Ford_Fiesta_1 ==
  Selector : Selector  IndexMode=Zero-based  IndexOptions=Starting index (dialog)  Indices=PortIndex  OutputSizes=PortWidth(1)
  U : Outport
  wires:
  Selector/1 -> U/1
  [SimCoreSensors] -> Selector/1

== system V2x_TC_IntersectionCollisionWarning_cs/SELF_Ford_FocusWagon_1 ==
  Selector : Selector  IndexMode=Zero-based  IndexOptions=Starting index (dialog)  Indices=PortIndex  OutputSizes=PortWidth(1)
  U : Outport
  wires:
  Selector/1 -> U/1
  [SimCoreSensors] -> Selector/1

== system V2x_TC_IntersectionCollisionWarning_cs/SELF_Male_Regular_wSuitcase_1 ==
  Selector : Selector  IndexMode=Zero-based  IndexOptions=Starting index (dialog)  Indices=PortIndex  OutputSizes=PortWidth(1)
  U : Outport
  wires:
  Selector/1 -> U/1
  [SimCoreSensors] -> Selector/1

== system V2x_TC_IntersectionCollisionWarning_cs/SELF_Mazda_RX8_1 ==
  Selector : Selector  IndexMode=Zero-based  IndexOptions=Starting index (dialog)  Indices=PortIndex  OutputSizes=PortWidth(1)
  U : Outport
  wires:
  Selector/1 -> U/1
  [SimCoreSensors] -> Selector/1

== system V2x_TC_IntersectionCollisionWarning_cs/SELF_Mazda_RX8_2 ==
  Selector : Selector  IndexMode=Zero-based  IndexOptions=Starting index (dialog)  Indices=PortIndex  OutputSizes=PortWidth(1)
  U : Outport
  wires:
  Selector/1 -> U/1
  [SimCoreSensors] -> Selector/1

== system V2x_TC_IntersectionCollisionWarning_cs/SELF_Sphere_1 ==
  Selector : Selector  IndexMode=Zero-based  IndexOptions=Starting index (dialog)  Indices=PortIndex  OutputSizes=PortWidth(1)
  U : Outport
  wires:
  Selector/1 -> U/1
  [SimCoreSensors] -> Selector/1

== system V2x_TC_IntersectionCollisionWarning_cs/SELF_Toyota_Previa_1 ==
  Selector : Selector  IndexMode=Zero-based  IndexOptions=Starting index (dialog)  Indices=PortIndex  OutputSizes=PortWidth(1)
  U : Outport
  wires:
  Selector/1 -> U/1
  [SimCoreSensors] -> Selector/1

== system V2x_TC_IntersectionCollisionWarning_cs/SELF_Toyota_Yaris_1 ==
  Selector : Selector  IndexMode=Zero-based  IndexOptions=Starting index (dialog)  Indices=PortIndex  OutputSizes=PortWidth(1)
  U : Outport
  wires:
  Selector/1 -> U/1
  [SimCoreSensors] -> Selector/1
